simulink model "All_64_Coils"  (R2021b)
settings: Solver=FixedStepAuto  StartTime=-3000e-6  StopTime=3000e-6  FixedStep=25e-6  RelTol=1e-3
(part 11 of 70)

== system All_64_Coils/Coil-3/Inner_5_coils/Square-4 ==
  Input : Inport
  Input1 : Inport  Port=2
  Input2 : Inport  Port=3
  Input3 : Inport  Port=4
  F_xx_4 : Display
  F_xz_4 : Display
  F_yy_4 : Display
  F_yz_4 : Display
  Scope : Scope  NumInputPorts=4
  Square - 4 : MATLAB Function  TreatAsAtomicUnit=on
  Output : Outport
  Output1 : Outport  Port=2
  Output2 : Outport  Port=3
  Output3 : Outport  Port=4
  wires:
  Input3/1 -> Square - 4/4(I)
  Input/1 -> Square - 4/1(x)
  Input1/1 -> Square - 4/2(y)
  Input2/1 -> Square - 4/3(z)
  Square - 4/3(F_yy_4) -> F_yy_4/1, Output2/1, Scope/3
  Square - 4/2(F_xz_4) -> Scope/2, F_xz_4/1, Output1/1
  Square - 4/4(F_yz_4) -> F_yz_4/1, Output3/1, Scope/4
  Square - 4/1(F_xx_4) -> F_xx_4/1, Output/1, Scope/1

== system All_64_Coils/Coil-3/Inner_5_coils/Square-5 ==
  Input : Inport
  Input1 : Inport  Port=2
  Input2 : Inport  Port=3
  Input3 : Inport  Port=4
  F_xx_5 : Display
  F_xz_5 : Display
  F_yy_5 : Display
  F_yz_5 : Display
  Scope : Scope  NumInputPorts=4
  Square - 5 : MATLAB Function  TreatAsAtomicUnit=on
  Output : Outport
  Output1 : Outport  Port=2
  Output2 : Outport  Port=3
  Output3 : Outport  Port=4
  wires:
  Input3/1 -> Square - 5/4(I)
  Input/1 -> Square - 5/1(x)
  Input1/1 -> Square - 5/2(y)
  Input2/1 -> Square - 5/3(z)
  Square - 5/3(F_yy_5) -> F_yy_5/1, Output2/1, Scope/3
  Square - 5/2(F_xz_5) -> F_xz_5/1, Output1/1, Scope/2
  Square - 5/4(F_yz_5) -> F_yz_5/1, Output3/1, Scope/4
  Square - 5/1(F_xx_5) -> F_xx_5/1, Output/1, Scope/1

== system All_64_Coils/Coil-3/Outer_5_Coils/Square-10 ==
  Input : Inport
  Input1 : Inport  Port=2
  Input2 : Inport  Port=3
  Input3 : Inport  Port=4
  F_xx_10 : Display
  F_xz_10 : Display
  F_yy_10 : Display
  F_yz_10 : Display
  Scope : Scope  NumInputPorts=4
  Square - 10 : MATLAB Function  TreatAsAtomicUnit=on
  Output : Outport
  Output1 : Outport  Port=2
  Output2 : Outport  Port=3
  Output3 : Outport  Port=4
  wires:
  Square - 10/1(F_xx_10) -> Output/1, F_xx_10/1, Scope/1
  Square - 10/4(F_yz_10) -> Output3/1, F_yz_10/1, Scope/4
  Square - 10/2(F_xz_10) -> Output1/1, F_xz_10/1, Scope/2
  Square - 10/3(F_yy_10) -> Output2/1, F_yy_10/1, Scope/3
  Input2/1 -> Square - 10/3(z)
  Input1/1 -> Square - 10/2(y)
  Input/1 -> Square - 10/1(x)
  Input3/1 -> Square - 10/4(I)

== system All_64_Coils/Coil-3/Outer_5_Coils/Square-6 ==
  Input : Inport
  Input1 : Inport  Port=2
  Input2 : Inport  Port=3
  Input3 : Inport  Port=4
  F_xx_6 : Display
  F_xz_6 : Display
  F_yy_6 : Display
  F_yz_6 : Display
  Scope : Scope  NumInputPorts=4
  Square - 6 : MATLAB Function  TreatAsAtomicUnit=on
  Output : Outport
  Output1 : Outport  Port=2
  Output2 : Outport  Port=3
  Output3 : Outport  Port=4
  wires:
  Square - 6/2(F_xz_6) -> Output1/1, F_xz_6/1, Scope/2
  Square - 6/3(F_yy_6) -> F_yy_6/1, Output2/1, Scope/3
  Square - 6/1(F_xx_6) -> Output/1, F_xx_6/1, Scope/1
  Square - 6/4(F_yz_6) -> Output3/1, F_yz_6/1, Scope/4
  Input/1 -> Square - 6/1(x)
  Input1/1 -> Square - 6/2(y)
  Input2/1 -> Square - 6/3(z)
  Input3/1 -> Square - 6/4(I)

== system All_64_Coils/Coil-3/Outer_5_Coils/Square-7 ==
  Input : Inport
  Input1 : Inport  Port=2
  Input2 : Inport  Port=3
  Input3 : Inport  Port=4
  F_xx_7 : Display
  F_xz_7 : Display
  F_yy_7 : Display
  F_yz_7 : Display
  Scope : Scope  NumInputPorts=4
  Square - 7 : MATLAB Function  TreatAsAtomicUnit=on
  Output : Outport
  Output1 : Outport  Port=2
  Output2 : Outport  Port=3
  Output3 : Outport  Port=4
  wires:
  Square - 7/1(F_xx_7) -> Output/1, F_xx_7/1, Scope/1
  Square - 7/2(F_xz_7) -> Output1/1, F_xz_7/1, Scope/2
  Square - 7/4(F_yz_7) -> Output3/1, F_yz_7/1, Scope/4
  Square - 7/3(F_yy_7) -> Output2/1, F_yy_7/1, Scope/3
  Input2/1 -> Square - 7/3(z)
  Input1/1 -> Square - 7/2(y)
  Input/1 -> Square - 7/1(x)
  Input3/1 -> Square - 7/4(I)

== system All_64_Coils/Coil-3/Outer_5_Coils/Square-8 ==
  Input : Inport
  Input1 : Inport  Port=2
  Input2 : Inport  Port=3
  Input3 : Inport  Port=4
  F_xx_8 : Display
  F_xz_8 : Display
  F_yy_8 : Display
  F_yz_8 : Display
  Scope : Scope  NumInputPorts=4
  Square - 8 : MATLAB Function  TreatAsAtomicUnit=on
  Output : Outport
  Output1 : Outport  Port=2
  Output2 : Outport  Port=3
  Output3 : Outport  Port=4
  wires:
  Square - 8/1(F_xx_8) -> Output/1, F_xx_8/1, Scope/1
  Square - 8/4(F_yz_8) -> Output3/1, F_yz_8/1, Scope/4
  Square - 8/2(F_xz_8) -> Output1/1, F_xz_8/1, Scope/2
  Square - 8/3(F_yy_8) -> F_yy_8/1, Output2/1, Scope/3
  Input2/1 -> Square - 8/3(z)
  Input1/1 -> Square - 8/2(y)
  Input/1 -> Square - 8/1(x)
  Input3/1 -> Square - 8/4(I)

== system All_64_Coils/Coil-3/Outer_5_Coils/Square-9 ==
  Input : Inport
  Input1 : Inport  Port=2
  Input2 : Inport  Port=3
  Input3 : Inport  Port=4
  F_xx_9 : Display
  F_xz_9 : Display
  F_yy_9 : Display
  F_yz_9 : Display
  Scope : Scope  NumInputPorts=4
  Square - 9 : MATLAB Function  TreatAsAtomicUnit=on
  Output : Outport
  Output1 : Outport  Port=2
  Output2 : Outport  Port=3
  Output3 : Outport  Port=4
  wires:
  Square - 9/1(F_xx_9) -> Output/1, F_xx_9/1, Scope/1
  Square - 9/4(F_yz_9) -> Output3/1, F_yz_9/1, Scope/4
  Square - 9/2(F_xz_9) -> Output1/1, F_xz_9/1, Scope/2
  Square - 9/3(F_yy_9) -> Output2/1, F_yy_9/1, Scope/3
  Input2/1 -> Square - 9/3(z)
  Input1/1 -> Square - 9/2(y)
  Input/1 -> Square - 9/1(x)
  Input3/1 -> Square - 9/4(I)

== system All_64_Coils/Coil-30/Inner_5_coils/Square-1 ==
  Input : Inport
  Input1 : Inport  Port=2
  Input2 : Inport  Port=3
  Input3 : Inport  Port=4
  F_xx_1 : Display
  F_xz_1 : Display
  F_yy_1 : Display
  F_yz_1 : Display
  Scope : Scope  NumInputPorts=4
  Square - 1 : MATLAB Function  TreatAsAtomicUnit=on
  Output : Outport
  Output1 : Outport  Port=2
  Output2 : Outport  Port=3
  Output3 : Outport  Port=4
  wires:
  Input3/1 -> Square - 1/4(I)
  Input2/1 -> Square - 1/3(z)
  Input1/1 -> Square - 1/2(y)
  Input/1 -> Square - 1/1(x)
  Square - 1/4(F_yz_1) -> F_yz_1/1, Output3/1, Scope/4
  Square - 1/1(F_xx_1) -> Scope/1, F_xx_1/1, Output/1
  Square - 1/3(F_yy_1) -> Scope/3, Output2/1, F_yy_1/1
  Square - 1/2(F_xz_1) -> Scope/2, F_xz_1/1, Output1/1

== system All_64_Coils/Coil-30/Inner_5_coils/Square-2 ==
  Input : Inport
  Input1 : Inport  Port=2
  Input2 : Inport  Port=3
  Input3 : Inport  Port=4
  F_xx_2 : Display
  F_xz_2 : Display
  F_yy_2 : Display
  F_yz_2 : Display
  Scope : Scope  NumInputPorts=4
  Square - 2 : MATLAB Function  TreatAsAtomicUnit=on
  Output : Outport
  Output1 : Outport  Port=2
  Output2 : Outport  Port=3
  Output3 : Outport  Port=4
  wires:
  Input3/1 -> Square - 2/4(I)
  Input/1 -> Square - 2/1(x)
  Input1/1 -> Square - 2/2(y)
  Input2/1 -> Square - 2/3(z)
  Square - 2/3(F_yy_2) -> F_yy_2/1, Output2/1, Scope/3
  Square - 2/4(F_yz_2) -> F_yz_2/1, Output3/1, Scope/4
  Square - 2/2(F_xz_2) -> F_xz_2/1, Output1/1, Scope/2
  Square - 2/1(F_xx_2) -> F_xx_2/1, Output/1, Scope/1

== system All_64_Coils/Coil-30/Inner_5_coils/Square-3 ==
  Input : Inport
  Input1 : Inport  Port=2
  Input2 : Inport  Port=3
  Input3 : Inport  Port=4
  F_xx_3 : Display
  F_xz_3 : Display
  F_yy_3 : Display
  F_yz_3 : Display
  Scope : Scope  NumInputPorts=4
  Square - 3 : MATLAB Function  TreatAsAtomicUnit=on
  Output : Outport
  Output1 : Outport  Port=2
  Output2 : Outport  Port=3
  Output3 : Outport  Port=4
  wires:
  Input3/1 -> Square - 3/4(I)
  Input/1 -> Square - 3/1(x)
  Input1/1 -> Square - 3/2(y)
  Input2/1 -> Square - 3/3(z)
  Square - 3/3(F_yy_3) -> Output2/1, F_yy_3/1, Scope/3
  Square - 3/2(F_xz_3) -> F_xz_3/1, Output1/1, Scope/2
  Square - 3/4(F_yz_3) -> F_yz_3/1, Output3/1, Scope/4
  Square - 3/1(F_xx_3) -> F_xx_3/1, Output/1, Scope/1

== system All_64_Coils/Coil-30/Inner_5_coils/Square-4 ==
  Input : Inport
  Input1 : Inport  Port=2
  Input2 : Inport  Port=3
  Input3 : Inport  Port=4
  F_xx_4 : Display
  F_xz_4 : Display
  F_yy_4 : Display
  F_yz_4 : Display
  Scope : Scope  NumInputPorts=4
  Square - 4 : MATLAB Function  TreatAsAtomicUnit=on
  Output : Outport
  Output1 : Outport  Port=2
  Output2 : Outport  Port=3
  Output3 : Outport  Port=4
  wires:
  Input3/1 -> Square - 4/4(I)
  Input/1 -> Square - 4/1(x)
  Input1/1 -> Square - 4/2(y)
  Input2/1 -> Square - 4/3(z)
  Square - 4/3(F_yy_4) -> F_yy_4/1, Output2/1, Scope/3
  Square - 4/2(F_xz_4) -> Scope/2, F_xz_4/1, Output1/1
  Square - 4/4(F_yz_4) -> F_yz_4/1, Output3/1, Scope/4
  Square - 4/1(F_xx_4) -> F_xx_4/1, Output/1, Scope/1

== system All_64_Coils/Coil-30/Inner_5_coils/Square-5 ==
  Input : Inport
  Input1 : Inport  Port=2
  Input2 : Inport  Port=3
  Input3 : Inport  Port=4
  F_xx_5 : Display
  F_xz_5 : Display
  F_yy_5 : Display
  F_yz_5 : Display
  Scope : Scope  NumInputPorts=4
  Square - 5 : MATLAB Function  TreatAsAtomicUnit=on
  Output : Outport
  Output1 : Outport  Port=2
  Output2 : Outport  Port=3
  Output3 : Outport  Port=4
  wires:
  Input3/1 -> Square - 5/4(I)
  Input/1 -> Square - 5/1(x)
  Input1/1 -> Square - 5/2(y)
  Input2/1 -> Square - 5/3(z)
  Square - 5/3(F_yy_5) -> F_yy_5/1, Output2/1, Scope/3
  Square - 5/2(F_xz_5) -> F_xz_5/1, Output1/1, Scope/2
  Square - 5/4(F_yz_5) -> F_yz_5/1, Output3/1, Scope/4
  Square - 5/1(F_xx_5) -> F_xx_5/1, Output/1, Scope/1

== system All_64_Coils/Coil-30/Outer_5_Coils/Square-10 ==
  Input : Inport
  Input1 : Inport  Port=2
  Input2 : Inport  Port=3
  Input3 : Inport  Port=4
  F_xx_10 : Display
  F_xz_10 : Display
  F_yy_10 : Display
  F_yz_10 : Display
  Scope : Scope  NumInputPorts=4
  Square - 10 : MATLAB Function  TreatAsAtomicUnit=on
  Output : Outport
  Output1 : Outport  Port=2
  Output2 : Outport  Port=3
  Output3 : Outport  Port=4
  wires:
  Square - 10/1(F_xx_10) -> Output/1, F_xx_10/1, Scope/1
  Square - 10/4(F_yz_10) -> Output3/1, F_yz_10/1, Scope/4
  Square - 10/2(F_xz_10) -> Output1/1, F_xz_10/1, Scope/2
  Square - 10/3(F_yy_10) -> Output2/1, F_yy_10/1, Scope/3
  Input2/1 -> Square - 10/3(z)
  Input1/1 -> Square - 10/2(y)
  Input/1 -> Square - 10/1(x)
  Input3/1 -> Square - 10/4(I)

== system All_64_Coils/Coil-30/Outer_5_Coils/Square-6 ==
  Input : Inport
  Input1 : Inport  Port=2
  Input2 : Inport  Port=3
  Input3 : Inport  Port=4
  F_xx_6 : Display
  F_xz_6 : Display
  F_yy_6 : Display
  F_yz_6 : Display
  Scope : Scope  NumInputPorts=4
  Square - 6 : MATLAB Function  TreatAsAtomicUnit=on
  Output : Outport
  Output1 : Outport  Port=2
  Output2 : Outport  Port=3
  Output3 : Outport  Port=4
  wires:
  Square - 6/2(F_xz_6) -> Output1/1, F_xz_6/1, Scope/2
  Square - 6/3(F_yy_6) -> F_yy_6/1, Output2/1, Scope/3
  Square - 6/1(F_xx_6) -> Output/1, F_xx_6/1, Scope/1
  Square - 6/4(F_yz_6) -> Output3/1, F_yz_6/1, Scope/4
  Input/1 -> Square - 6/1(x)
  Input1/1 -> Square - 6/2(y)
  Input2/1 -> Square - 6/3(z)
  Input3/1 -> Square - 6/4(I)

== system All_64_Coils/Coil-30/Outer_5_Coils/Square-7 ==
  Input : Inport
  Input1 : Inport  Port=2
  Input2 : Inport  Port=3
  Input3 : Inport  Port=4
  F_xx_7 : Display
  F_xz_7 : Display
  F_yy_7 : Display
  F_yz_7 : Display
  Scope : Scope  NumInputPorts=4
  Square - 7 : MATLAB Function  TreatAsAtomicUnit=on
  Output : Outport
  Output1 : Outport  Port=2
  Output2 : Outport  Port=3
  Output3 : Outport  Port=4
  wires:
  Square - 7/1(F_xx_7) -> Output/1, F_xx_7/1, Scope/1
  Square - 7/2(F_xz_7) -> Output1/1, F_xz_7/1, Scope/2
  Square - 7/4(F_yz_7) -> Output3/1, F_yz_7/1, Scope/4
  Square - 7/3(F_yy_7) -> Output2/1, F_yy_7/1, Scope/3
  Input2/1 -> Square - 7/3(z)
  Input1/1 -> Square - 7/2(y)
  Input/1 -> Square - 7/1(x)
  Input3/1 -> Square - 7/4(I)

== system All_64_Coils/Coil-30/Outer_5_Coils/Square-8 ==
  Input : Inport
  Input1 : Inport  Port=2
  Input2 : Inport  Port=3
  Input3 : Inport  Port=4
  F_xx_8 : Display
  F_xz_8 : Display
  F_yy_8 : Display
  F_yz_8 : Display
  Scope : Scope  NumInputPorts=4
  Square - 8 : MATLAB Function  TreatAsAtomicUnit=on
  Output : Outport
  Output1 : Outport  Port=2
  Output2 : Outport  Port=3
  Output3 : Outport  Port=4
  wires:
  Square - 8/1(F_xx_8) -> Output/1, F_xx_8/1, Scope/1
  Square - 8/4(F_yz_8) -> Output3/1, F_yz_8/1, Scope/4
  Square - 8/2(F_xz_8) -> Output1/1, F_xz_8/1, Scope/2
  Square - 8/3(F_yy_8) -> F_yy_8/1, Output2/1, Scope/3
  Input2/1 -> Square - 8/3(z)
  Input1/1 -> Square - 8/2(y)
  Input/1 -> Square - 8/1(x)
  Input3/1 -> Square - 8/4(I)

== system All_64_Coils/Coil-30/Outer_5_Coils/Square-9 ==
  Input : Inport
  Input1 : Inport  Port=2
  Input2 : Inport  Port=3
  Input3 : Inport  Port=4
  F_xx_9 : Display
  F_xz_9 : Display
  F_yy_9 : Display
  F_yz_9 : Display
  Scope : Scope  NumInputPorts=4
  Square - 9 : MATLAB Function  TreatAsAtomicUnit=on
  Output : Outport
  Output1 : Outport  Port=2
  Output2 : Outport  Port=3
  Output3 : Outport  Port=4
  wires:
  Square - 9/1(F_xx_9) -> Output/1, F_xx_9/1, Scope/1
  Square - 9/4(F_yz_9) -> Output3/1, F_yz_9/1, Scope/4
  Square - 9/2(F_xz_9) -> Output1/1, F_xz_9/1, Scope/2
  Square - 9/3(F_yy_9) -> Output2/1, F_yy_9/1, Scope/3
  Input2/1 -> Square - 9/3(z)
  Input1/1 -> Square - 9/2(y)
  Input/1 -> Square - 9/1(x)
  Input3/1 -> Square - 9/4(I)

== system All_64_Coils/Coil-31/Inner_5_coils/Square-1 ==
  Input : Inport
  Input1 : Inport  Port=2
  Input2 : Inport  Port=3
  Input3 : Inport  Port=4
  F_xx_1 : Display
  F_xz_1 : Display
  F_yy_1 : Display
  F_yz_1 : Display
  Scope : Scope  NumInputPorts=4
  Square - 1 : MATLAB Function  TreatAsAtomicUnit=on
  Output : Outport
  Output1 : Outport  Port=2
  Output2 : Outport  Port=3
  Output3 : Outport  Port=4
  wires:
  Square - 1/2(F_xz_1) -> Output1/1, F_xz_1/1, Scope/2
  Square - 1/3(F_yy_1) -> F_yy_1/1, Output2/1, Scope/3
  Square - 1/1(F_xx_1) -> Output/1, F_xx_1/1, Scope/1
  Square - 1/4(F_yz_1) -> Scope/4, Output3/1, F_yz_1/1
  Input/1 -> Square - 1/1(x)
  Input1/1 -> Square - 1/2(y)
  Input2/1 -> Square - 1/3(z)
  Input3/1 -> Square - 1/4(I)

== system All_64_Coils/Coil-31/Inner_5_coils/Square-2 ==
  Input : Inport
  Input1 : Inport  Port=2
  Input2 : Inport  Port=3
  Input3 : Inport  Port=4
  F_xx_2 : Display
  F_xz_2 : Display
  F_yy_2 : Display
  F_yz_2 : Display
  Scope : Scope  NumInputPorts=4
  Square - 2 : MATLAB Function  TreatAsAtomicUnit=on
  Output : Outport
  Output1 : Outport  Port=2
  Output2 : Outport  Port=3
  Output3 : Outport  Port=4
  wires:
  Square - 2/1(F_xx_2) -> Scope/1, Output/1, F_xx_2/1
  Square - 2/2(F_xz_2) -> Scope/2, Output1/1, F_xz_2/1
  Square - 2/4(F_yz_2) -> Scope/4, Output3/1, F_yz_2/1
  Square - 2/3(F_yy_2) -> Scope/3, Output2/1, F_yy_2/1
  Input2/1 -> Square - 2/3(z)
  Input1/1 -> Square - 2/2(y)
  Input/1 -> Square - 2/1(x)
  Input3/1 -> Square - 2/4(I)

== system All_64_Coils/Coil-31/Inner_5_coils/Square-3 ==
  Input : Inport
  Input1 : Inport  Port=2
  Input2 : Inport  Port=3
  Input3 : Inport  Port=4
  F_xx_3 : Display
  F_xz_3 : Display
  F_yy_3 : Display
  F_yz_3 : Display
  Scope : Scope  NumInputPorts=4
  Square - 3 : MATLAB Function  TreatAsAtomicUnit=on
  Output : Outport
  Output1 : Outport  Port=2
  Output2 : Outport  Port=3
  Output3 : Outport  Port=4
  wires:
  Square - 3/1(F_xx_3) -> Scope/1, Output/1, F_xx_3/1
  Square - 3/4(F_yz_3) -> Scope/4, Output3/1, F_yz_3/1
  Square - 3/2(F_xz_3) -> Scope/2, Output1/1, F_xz_3/1
  Square - 3/3(F_yy_3) -> Scope/3, F_yy_3/1, Output2/1
  Input2/1 -> Square - 3/3(z)
  Input1/1 -> Square - 3/2(y)
  Input/1 -> Square - 3/1(x)
  Input3/1 -> Square - 3/4(I)

== system All_64_Coils/Coil-31/Inner_5_coils/Square-4 ==
  Input : Inport
  Input1 : Inport  Port=2
  Input2 : Inport  Port=3
  Input3 : Inport  Port=4
  F_xx_4 : Display
  F_xz_4 : Display
  F_yy_4 : Display
  F_yz_4 : Display
  Scope : Scope  NumInputPorts=4
  Square - 4 : MATLAB Function  TreatAsAtomicUnit=on
  Output : Outport
  Output1 : Outport  Port=2
  Output2 : Outport  Port=3
  Output3 : Outport  Port=4
  wires:
  Square - 4/1(F_xx_4) -> Scope/1, Output/1, F_xx_4/1
  Square - 4/4(F_yz_4) -> Scope/4, Output3/1, F_yz_4/1
  Square - 4/2(F_xz_4) -> Output1/1, F_xz_4/1, Scope/2
  Square - 4/3(F_yy_4) -> Scope/3, Output2/1, F_yy_4/1
  Input2/1 -> Square - 4/3(z)
  Input1/1 -> Square - 4/2(y)
  Input/1 -> Square - 4/1(x)
  Input3/1 -> Square - 4/4(I)

== system All_64_Coils/Coil-31/Inner_5_coils/Square-5 ==
  Input : Inport
  Input1 : Inport  Port=2
  Input2 : Inport  Port=3
  Input3 : Inport  Port=4
  F_xx_5 : Display
  F_xz_5 : Display
  F_yy_5 : Display
  F_yz_5 : Display
  Scope : Scope  NumInputPorts=4
  Square - 5 : MATLAB Function  TreatAsAtomicUnit=on
  Output : Outport
  Output1 : Outport  Port=2
  Output2 : Outport  Port=3
  Output3 : Outport  Port=4
  wires:
  Square - 5/1(F_xx_5) -> Scope/1, Output/1, F_xx_5/1
  Square - 5/4(F_yz_5) -> Scope/4, Output3/1, F_yz_5/1
  Square - 5/2(F_xz_5) -> Scope/2, Output1/1, F_xz_5/1
  Square - 5/3(F_yy_5) -> Scope/3, Output2/1, F_yy_5/1
  Input2/1 -> Square - 5/3(z)
  Input1/1 -> Square - 5/2(y)
  Input/1 -> Square - 5/1(x)
  Input3/1 -> Square - 5/4(I)

== system All_64_Coils/Coil-31/Outer_5_Coils/Square-10 ==
  Input : Inport
  Input1 : Inport  Port=2
  Input2 : Inport  Port=3
  Input3 : Inport  Port=4
  F_xx_10 : Display
  F_xz_10 : Display
  F_yy_10 : Display
  F_yz_10 : Display
  Scope : Scope  NumInputPorts=4
  Square - 10 : MATLAB Function  TreatAsAtomicUnit=on
  Output : Outport
  Output1 : Outport  Port=2
  Output2 : Outport  Port=3
  Output3 : Outport  Port=4
  wires:
  Input3/1 -> Square - 10/4(I)
  Input/1 -> Square - 10/1(x)
  Input1/1 -> Square - 10/2(y)
  Input2/1 -> Square - 10/3(z)
  Square - 10/3(F_yy_10) -> Scope/3, F_yy_10/1, Output2/1
  Square - 10/2(F_xz_10) -> Scope/2, F_xz_10/1, Output1/1
  Square - 10/4(F_yz_10) -> Scope/4, F_yz_10/1, Output3/1
  Square - 10/1(F_xx_10) -> Scope/1, F_xx_10/1, Output/1

== system All_64_Coils/Coil-31/Outer_5_Coils/Square-6 ==
  Input : Inport
  Input1 : Inport  Port=2
  Input2 : Inport  Port=3
  Input3 : Inport  Port=4
  F_xx_6 : Display
  F_xz_6 : Display
  F_yy_6 : Display
  F_yz_6 : Display
  Scope : Scope  NumInputPorts=4
  Square - 6 : MATLAB Function  TreatAsAtomicUnit=on
  Output : Outport
  Output1 : Outport  Port=2
  Output2 : Outport  Port=3
  Output3 : Outport  Port=4
  wires:
  Input3/1 -> Square - 6/4(I)
  Input2/1 -> Square - 6/3(z)
  Input1/1 -> Square - 6/2(y)
  Input/1 -> Square - 6/1(x)
  Square - 6/4(F_yz_6) -> Scope/4, F_yz_6/1, Output3/1
  Square - 6/1(F_xx_6) -> Scope/1, F_xx_6/1, Output/1
  Square - 6/3(F_yy_6) -> Scope/3, Output2/1, F_yy_6/1
  Square - 6/2(F_xz_6) -> Scope/2, F_xz_6/1, Output1/1

== system All_64_Coils/Coil-31/Outer_5_Coils/Square-7 ==
  Input : Inport
  Input1 : Inport  Port=2
  Input2 : Inport  Port=3
  Input3 : Inport  Port=4
  F_xx_7 : Display
  F_xz_7 : Display
  F_yy_7 : Display
  F_yz_7 : Display
  Scope : Scope  NumInputPorts=4
  Square - 7 : MATLAB Function  TreatAsAtomicUnit=on
  Output : Outport
  Output1 : Outport  Port=2
  Output2 : Outport  Port=3
  Output3 : Outport  Port=4
  wires:
  Input3/1 -> Square - 7/4(I)
  Input/1 -> Square - 7/1(x)
  Input1/1 -> Square - 7/2(y)
  Input2/1 -> Square - 7/3(z)
  Square - 7/3(F_yy_7) -> Scope/3, F_yy_7/1, Output2/1
  Square - 7/4(F_yz_7) -> Scope/4, F_yz_7/1, Output3/1
  Square - 7/2(F_xz_7) -> Scope/2, F_xz_7/1, Output1/1
  Square - 7/1(F_xx_7) -> Scope/1, F_xx_7/1, Output/1

== system All_64_Coils/Coil-31/Outer_5_Coils/Square-8 ==
  Input : Inport
  Input1 : Inport  Port=2
  Input2 : Inport  Port=3
  Input3 : Inport  Port=4
  F_xx_8 : Display
  F_xz_8 : Display
  F_yy_8 : Display
  F_yz_8 : Display
  Scope : Scope  NumInputPorts=4
  Square - 8 : MATLAB Function  TreatAsAtomicUnit=on
  Output : Outport
  Output1 : Outport  Port=2
  Output2 : Outport  Port=3
  Output3 : Outport  Port=4
  wires:
  Input3/1 -> Square - 8/4(I)
  Input/1 -> Square - 8/1(x)
  Input1/1 -> Square - 8/2(y)
  Input2/1 -> Square - 8/3(z)
  Square - 8/3(F_yy_8) -> Scope/3, Output2/1, F_yy_8/1
  Square - 8/2(F_xz_8) -> Scope/2, F_xz_8/1, Output1/1
  Square - 8/4(F_yz_8) -> Scope/4, F_yz_8/1, Output3/1
  Square - 8/1(F_xx_8) -> Scope/1, F_xx_8/1, Output/1

== system All_64_Coils/Coil-31/Outer_5_Coils/Square-9 ==
  Input : Inport
  Input1 : Inport  Port=2
  Input2 : Inport  Port=3
  Input3 : Inport  Port=4
  F_xx_9 : Display
  F_xz_9 : Display
  F_yy_9 : Display
  F_yz_9 : Display
  Scope : Scope  NumInputPorts=4
  Square - 9 : MATLAB Function  TreatAsAtomicUnit=on
  Output : Outport
  Output1 : Outport  Port=2
  Output2 : Outport  Port=3
  Output3 : Outport  Port=4
  wires:
  Input3/1 -> Square - 9/4(I)
  Input/1 -> Square - 9/1(x)
  Input1/1 -> Square - 9/2(y)
  Input2/1 -> Square - 9/3(z)
  Square - 9/3(F_yy_9) -> Scope/3, F_yy_9/1, Output2/1
  Square - 9/2(F_xz_9) -> Scope/2, F_xz_9/1, Output1/1
  Square - 9/4(F_yz_9) -> Scope/4, F_yz_9/1, Output3/1
  Square - 9/1(F_xx_9) -> Scope/1, F_xx_9/1, Output/1

== system All_64_Coils/Coil-32/Inner_5_coils/Square-1 ==
  Input : Inport
  Input1 : Inport  Port=2
  Input2 : Inport  Port=3
  Input3 : Inport  Port=4
  F_xx_1 : Display
  F_xz_1 : Display
  F_yy_1 : Display
  F_yz_1 : Display
  Scope : Scope  NumInputPorts=4
  Square - 1 : MATLAB Function  TreatAsAtomicUnit=on
  Output : Outport
  Output1 : Outport  Port=2
  Output2 : Outport  Port=3
  Output3 : Outport  Port=4
  wires:
  Square - 1/2(F_xz_1) -> Output1/1, F_xz_1/1, Scope/2
  Square - 1/3(F_yy_1) -> F_yy_1/1, Output2/1, Scope/3
  Square - 1/1(F_xx_1) -> Output/1, F_xx_1/1, Scope/1
  Square - 1/4(F_yz_1) -> Scope/4, Output3/1, F_yz_1/1
  Input/1 -> Square - 1/1(x)
  Input1/1 -> Square - 1/2(y)
  Input2/1 -> Square - 1/3(z)
  Input3/1 -> Square - 1/4(I)

== system All_64_Coils/Coil-32/Inner_5_coils/Square-2 ==
  Input : Inport
  Input1 : Inport  Port=2
  Input2 : Inport  Port=3
  Input3 : Inport  Port=4
  F_xx_2 : Display
  F_xz_2 : Display
  F_yy_2 : Display
  F_yz_2 : Display
  Scope : Scope  NumInputPorts=4
  Square - 2 : MATLAB Function  TreatAsAtomicUnit=on
  Output : Outport
  Output1 : Outport  Port=2
  Output2 : Outport  Port=3
  Output3 : Outport  Port=4
  wires:
  Square - 2/1(F_xx_2) -> Scope/1, Output/1, F_xx_2/1
  Square - 2/2(F_xz_2) -> Scope/2, Output1/1, F_xz_2/1
  Square - 2/4(F_yz_2) -> Scope/4, Output3/1, F_yz_2/1
  Square - 2/3(F_yy_2) -> Scope/3, Output2/1, F_yy_2/1
  Input2/1 -> Square - 2/3(z)
  Input1/1 -> Square - 2/2(y)
  Input/1 -> Square - 2/1(x)
  Input3/1 -> Square - 2/4(I)

== system All_64_Coils/Coil-32/Inner_5_coils/Square-3 ==
  Input : Inport
  Input1 : Inport  Port=2
  Input2 : Inport  Port=3
  Input3 : Inport  Port=4
  F_xx_3 : Display
  F_xz_3 : Display
  F_yy_3 : Display
  F_yz_3 : Display
  Scope : Scope  NumInputPorts=4
  Square - 3 : MATLAB Function  TreatAsAtomicUnit=on
  Output : Outport
  Output1 : Outport  Port=2
  Output2 : Outport  Port=3
  Output3 : Outport  Port=4
  wires:
  Square - 3/1(F_xx_3) -> Scope/1, Output/1, F_xx_3/1
  Square - 3/4(F_yz_3) -> Scope/4, Output3/1, F_yz_3/1
  Square - 3/2(F_xz_3) -> Scope/2, Output1/1, F_xz_3/1
  Square - 3/3(F_yy_3) -> Scope/3, F_yy_3/1, Output2/1
  Input2/1 -> Square - 3/3(z)
  Input1/1 -> Square - 3/2(y)
  Input/1 -> Square - 3/1(x)
  Input3/1 -> Square - 3/4(I)

== system All_64_Coils/Coil-32/Inner_5_coils/Square-4 ==
  Input : Inport
  Input1 : Inport  Port=2
  Input2 : Inport  Port=3
  Input3 : Inport  Port=4
  F_xx_4 : Display
  F_xz_4 : Display
  F_yy_4 : Display
  F_yz_4 : Display
  Scope : Scope  NumInputPorts=4
  Square - 4 : MATLAB Function  TreatAsAtomicUnit=on
  Output : Outport
  Output1 : Outport  Port=2
  Output2 : Outport  Port=3
  Output3 : Outport  Port=4
  wires:
  Square - 4/1(F_xx_4) -> Scope/1, Output/1, F_xx_4/1
  Square - 4/4(F_yz_4) -> Scope/4, Output3/1, F_yz_4/1
  Square - 4/2(F_xz_4) -> Output1/1, F_xz_4/1, Scope/2
  Square - 4/3(F_yy_4) -> Scope/3, Output2/1, F_yy_4/1
  Input2/1 -> Square - 4/3(z)
  Input1/1 -> Square - 4/2(y)
  Input/1 -> Square - 4/1(x)
  Input3/1 -> Square - 4/4(I)

== system All_64_Coils/Coil-32/Inner_5_coils/Square-5 ==
  Input : Inport
  Input1 : Inport  Port=2
  Input2 : Inport  Port=3
  Input3 : Inport  Port=4
  F_xx_5 : Display
  F_xz_5 : Display
  F_yy_5 : Display
  F_yz_5 : Display
  Scope : Scope  NumInputPorts=4
  Square - 5 : MATLAB Function  TreatAsAtomicUnit=on
  Output : Outport
  Output1 : Outport  Port=2
  Output2 : Outport  Port=3
  Output3 : Outport  Port=4
  wires:
  Square - 5/1(F_xx_5) -> Scope/1, Output/1, F_xx_5/1
  Square - 5/4(F_yz_5) -> Scope/4, Output3/1, F_yz_5/1
  Square - 5/2(F_xz_5) -> Scope/2, Output1/1, F_xz_5/1
  Square - 5/3(F_yy_5) -> Scope/3, Output2/1, F_yy_5/1
  Input2/1 -> Square - 5/3(z)
  Input1/1 -> Square - 5/2(y)
  Input/1 -> Square - 5/1(x)
  Input3/1 -> Square - 5/4(I)

== system All_64_Coils/Coil-32/Outer_5_Coils/Square-10 ==
  Input : Inport
  Input1 : Inport  Port=2
  Input2 : Inport  Port=3
  Input3 : Inport  Port=4
  F_xx_10 : Display
  F_xz_10 : Display
  F_yy_10 : Display
  F_yz_10 : Display
  Scope : Scope  NumInputPorts=4
  Square - 10 : MATLAB Function  TreatAsAtomicUnit=on
  Output : Outport
  Output1 : Outport  Port=2
  Output2 : Outport  Port=3
  Output3 : Outport  Port=4
  wires:
  Input3/1 -> Square - 10/4(I)
  Input/1 -> Square - 10/1(x)
  Input1/1 -> Square - 10/2(y)
  Input2/1 -> Square - 10/3(z)
  Square - 10/3(F_yy_10) -> Scope/3, F_yy_10/1, Output2/1
  Square - 10/2(F_xz_10) -> Scope/2, F_xz_10/1, Output1/1
  Square - 10/4(F_yz_10) -> Scope/4, F_yz_10/1, Output3/1
  Square - 10/1(F_xx_10) -> Scope/1, F_xx_10/1, Output/1

== system All_64_Coils/Coil-32/Outer_5_Coils/Square-6 ==
  Input : Inport
  Input1 : Inport  Port=2
  Input2 : Inport  Port=3
  Input3 : Inport  Port=4
  F_xx_6 : Display
  F_xz_6 : Display
  F_yy_6 : Display
  F_yz_6 : Display
  Scope : Scope  NumInputPorts=4
  Square - 6 : MATLAB Function  TreatAsAtomicUnit=on
  Output : Outport
  Output1 : Outport  Port=2
  Output2 : Outport  Port=3
  Output3 : Outport  Port=4
  wires:
  Input3/1 -> Square - 6/4(I)
  Input2/1 -> Square - 6/3(z)
  Input1/1 -> Square - 6/2(y)
  Input/1 -> Square - 6/1(x)
  Square - 6/4(F_yz_6) -> Scope/4, F_yz_6/1, Output3/1
  Square - 6/1(F_xx_6) -> Scope/1, F_xx_6/1, Output/1
  Square - 6/3(F_yy_6) -> Scope/3, Output2/1, F_yy_6/1
  Square - 6/2(F_xz_6) -> Scope/2, F_xz_6/1, Output1/1

== system All_64_Coils/Coil-32/Outer_5_Coils/Square-7 ==
  Input : Inport
  Input1 : Inport  Port=2
  Input2 : Inport  Port=3
  Input3 : Inport  Port=4
  F_xx_7 : Display
  F_xz_7 : Display
  F_yy_7 : Display
  F_yz_7 : Display
  Scope : Scope  NumInputPorts=4
  Square - 7 : MATLAB Function  TreatAsAtomicUnit=on
  Output : Outport
  Output1 : Outport  Port=2
  Output2 : Outport  Port=3
  Output3 : Outport  Port=4
  wires:
  Input3/1 -> Square - 7/4(I)
  Input/1 -> Square - 7/1(x)
  Input1/1 -> Square - 7/2(y)
  Input2/1 -> Square - 7/3(z)
  Square - 7/3(F_yy_7) -> Scope/3, F_yy_7/1, Output2/1
  Square - 7/4(F_yz_7) -> Scope/4, F_yz_7/1, Output3/1
  Square - 7/2(F_xz_7) -> Scope/2, F_xz_7/1, Output1/1
  Square - 7/1(F_xx_7) -> Scope/1, F_xx_7/1, Output/1

== system All_64_Coils/Coil-32/Outer_5_Coils/Square-8 ==
  Input : Inport
  Input1 : Inport  Port=2
  Input2 : Inport  Port=3
  Input3 : Inport  Port=4
  F_xx_8 : Display
  F_xz_8 : Display
  F_yy_8 : Display
  F_yz_8 : Display
  Scope : Scope  NumInputPorts=4
  Square - 8 : MATLAB Function  TreatAsAtomicUnit=on
  Output : Outport
  Output1 : Outport  Port=2
  Output2 : Outport  Port=3
  Output3 : Outport  Port=4
  wires:
  Input3/1 -> Square - 8/4(I)
  Input/1 -> Square - 8/1(x)
  Input1/1 -> Square - 8/2(y)
  Input2/1 -> Square - 8/3(z)
  Square - 8/3(F_yy_8) -> Scope/3, Output2/1, F_yy_8/1
  Square - 8/2(F_xz_8) -> Scope/2, F_xz_8/1, Output1/1
  Square - 8/4(F_yz_8) -> Scope/4, F_yz_8/1, Output3/1
  Square - 8/1(F_xx_8) -> Scope/1, F_xx_8/1, Output/1

== system All_64_Coils/Coil-32/Outer_5_Coils/Square-9 ==
  Input : Inport
  Input1 : Inport  Port=2
  Input2 : Inport  Port=3
  Input3 : Inport  Port=4
  F_xx_9 : Display
  F_xz_9 : Display
  F_yy_9 : Display
  F_yz_9 : Display
  Scope : Scope  NumInputPorts=4
  Square - 9 : MATLAB Function  TreatAsAtomicUnit=on
  Output : Outport
  Output1 : Outport  Port=2
  Output2 : Outport  Port=3
  Output3 : Outport  Port=4
  wires:
  Input3/1 -> Square - 9/4(I)
  Input/1 -> Square - 9/1(x)
  Input1/1 -> Square - 9/2(y)
  Input2/1 -> Square - 9/3(z)
  Square - 9/3(F_yy_9) -> Scope/3, F_yy_9/1, Output2/1
  Square - 9/2(F_xz_9) -> Scope/2, F_xz_9/1, Output1/1
  Square - 9/4(F_yz_9) -> Scope/4, F_yz_9/1, Output3/1
  Square - 9/1(F_xx_9) -> Scope/1, F_xx_9/1, Output/1

== system All_64_Coils/Coil-33/Inner_5_coils/Square-1 ==
  Input : Inport
  Input1 : Inport  Port=2
  Input2 : Inport  Port=3
  Input3 : Inport  Port=4
  F_xx_1 : Display
  F_xz_1 : Display
  F_yy_1 : Display
  F_yz_1 : Display
  Scope : Scope  NumInputPorts=4
  Square - 1 : MATLAB Function  TreatAsAtomicUnit=on
  Output : Outport
  Output1 : Outport  Port=2
  Output2 : Outport  Port=3
  Output3 : Outport  Port=4
  wires:
  Input3/1 -> Square - 1/4(I)
  Input2/1 -> Square - 1/3(z)
  Input1/1 -> Square - 1/2(y)
  Input/1 -> Square - 1/1(x)
  Square - 1/4(F_yz_1) -> F_yz_1/1, Output3/1, Scope/4
  Square - 1/1(F_xx_1) -> Scope/1, F_xx_1/1, Output/1
  Square - 1/3(F_yy_1) -> Scope/3, Output2/1, F_yy_1/1
  Square - 1/2(F_xz_1) -> Scope/2, F_xz_1/1, Output1/1

== system All_64_Coils/Coil-33/Inner_5_coils/Square-2 ==
  Input : Inport
  Input1 : Inport  Port=2
  Input2 : Inport  Port=3
  Input3 : Inport  Port=4
  F_xx_2 : Display
  F_xz_2 : Display
  F_yy_2 : Display
  F_yz_2 : Display
  Scope : Scope  NumInputPorts=4
  Square - 2 : MATLAB Function  TreatAsAtomicUnit=on
  Output : Outport
  Output1 : Outport  Port=2
  Output2 : Outport  Port=3
  Output3 : Outport  Port=4
  wires:
  Input3/1 -> Square - 2/4(I)
  Input/1 -> Square - 2/1(x)
  Input1/1 -> Square - 2/2(y)
  Input2/1 -> Square - 2/3(z)
  Square - 2/3(F_yy_2) -> F_yy_2/1, Output2/1, Scope/3
  Square - 2/4(F_yz_2) -> F_yz_2/1, Output3/1, Scope/4
  Square - 2/2(F_xz_2) -> F_xz_2/1, Output1/1, Scope/2
  Square - 2/1(F_xx_2) -> F_xx_2/1, Output/1, Scope/1

== system All_64_Coils/Coil-33/Inner_5_coils/Square-3 ==
  Input : Inport
  Input1 : Inport  Port=2
  Input2 : Inport  Port=3
  Input3 : Inport  Port=4
  F_xx_3 : Display
  F_xz_3 : Display
  F_yy_3 : Display
  F_yz_3 : Display
  Scope : Scope  NumInputPorts=4
  Square - 3 : MATLAB Function  TreatAsAtomicUnit=on
  Output : Outport
  Output1 : Outport  Port=2
  Output2 : Outport  Port=3
  Output3 : Outport  Port=4
  wires:
  Input3/1 -> Square - 3/4(I)
  Input/1 -> Square - 3/1(x)
  Input1/1 -> Square - 3/2(y)
  Input2/1 -> Square - 3/3(z)
  Square - 3/3(F_yy_3) -> Output2/1, F_yy_3/1, Scope/3
  Square - 3/2(F_xz_3) -> F_xz_3/1, Output1/1, Scope/2
  Square - 3/4(F_yz_3) -> F_yz_3/1, Output3/1, Scope/4
  Square - 3/1(F_xx_3) -> F_xx_3/1, Output/1, Scope/1

== system All_64_Coils/Coil-33/Inner_5_coils/Square-4 ==
  Input : Inport
  Input1 : Inport  Port=2
  Input2 : Inport  Port=3
  Input3 : Inport  Port=4
  F_xx_4 : Display
  F_xz_4 : Display
  F_yy_4 : Display
  F_yz_4 : Display
  Scope : Scope  NumInputPorts=4
  Square - 4 : MATLAB Function  TreatAsAtomicUnit=on
  Output : Outport
  Output1 : Outport  Port=2
  Output2 : Outport  Port=3
  Output3 : Outport  Port=4
  wires:
  Input3/1 -> Square - 4/4(I)
  Input/1 -> Square - 4/1(x)
  Input1/1 -> Square - 4/2(y)
  Input2/1 -> Square - 4/3(z)
  Square - 4/3(F_yy_4) -> F_yy_4/1, Output2/1, Scope/3
  Square - 4/2(F_xz_4) -> Scope/2, F_xz_4/1, Output1/1
  Square - 4/4(F_yz_4) -> F_yz_4/1, Output3/1, Scope/4
  Square - 4/1(F_xx_4) -> F_xx_4/1, Output/1, Scope/1

== system All_64_Coils/Coil-33/Inner_5_coils/Square-5 ==
  Input : Inport
  Input1 : Inport  Port=2
  Input2 : Inport  Port=3
  Input3 : Inport  Port=4
  F_xx_5 : Display
  F_xz_5 : Display
  F_yy_5 : Display
  F_yz_5 : Display
  Scope : Scope  NumInputPorts=4
  Square - 5 : MATLAB Function  TreatAsAtomicUnit=on
  Output : Outport
  Output1 : Outport  Port=2
  Output2 : Outport  Port=3
  Output3 : Outport  Port=4
  wires:
  Input3/1 -> Square - 5/4(I)
  Input/1 -> Square - 5/1(x)
  Input1/1 -> Square - 5/2(y)
  Input2/1 -> Square - 5/3(z)
  Square - 5/3(F_yy_5) -> F_yy_5/1, Output2/1, Scope/3
  Square - 5/2(F_xz_5) -> F_xz_5/1, Output1/1, Scope/2
  Square - 5/4(F_yz_5) -> F_yz_5/1, Output3/1, Scope/4
  Square - 5/1(F_xx_5) -> F_xx_5/1, Output/1, Scope/1

== system All_64_Coils/Coil-33/Outer_5_Coils/Square-10 ==
  Input : Inport
  Input1 : Inport  Port=2
  Input2 : Inport  Port=3
  Input3 : Inport  Port=4
  F_xx_10 : Display
  F_xz_10 : Display
  F_yy_10 : Display
  F_yz_10 : Display
  Scope : Scope  NumInputPorts=4
  Square - 10 : MATLAB Function  TreatAsAtomicUnit=on
  Output : Outport
  Output1 : Outport  Port=2
  Output2 : Outport  Port=3
  Output3 : Outport  Port=4
  wires:
  Square - 10/1(F_xx_10) -> Output/1, F_xx_10/1, Scope/1
  Square - 10/4(F_yz_10) -> Output3/1, F_yz_10/1, Scope/4
  Square - 10/2(F_xz_10) -> Output1/1, F_xz_10/1, Scope/2
  Square - 10/3(F_yy_10) -> Output2/1, F_yy_10/1, Scope/3
  Input2/1 -> Square - 10/3(z)
  Input1/1 -> Square - 10/2(y)
  Input/1 -> Square - 10/1(x)
  Input3/1 -> Square - 10/4(I)

== system All_64_Coils/Coil-33/Outer_5_Coils/Square-6 ==
  Input : Inport
  Input1 : Inport  Port=2
  Input2 : Inport  Port=3
  Input3 : Inport  Port=4
  F_xx_6 : Display
  F_xz_6 : Display
  F_yy_6 : Display
  F_yz_6 : Display
  Scope : Scope  NumInputPorts=4
  Square - 6 : MATLAB Function  TreatAsAtomicUnit=on
  Output : Outport
  Output1 : Outport  Port=2
  Output2 : Outport  Port=3
  Output3 : Outport  Port=4
  wires:
  Square - 6/2(F_xz_6) -> Output1/1, F_xz_6/1, Scope/2
  Square - 6/3(F_yy_6) -> F_yy_6/1, Output2/1, Scope/3
  Square - 6/1(F_xx_6) -> Output/1, F_xx_6/1, Scope/1
  Square - 6/4(F_yz_6) -> Output3/1, F_yz_6/1, Scope/4
  Input/1 -> Square - 6/1(x)
  Input1/1 -> Square - 6/2(y)
  Input2/1 -> Square - 6/3(z)
  Input3/1 -> Square - 6/4(I)

== system All_64_Coils/Coil-33/Outer_5_Coils/Square-7 ==
  Input : Inport
  Input1 : Inport  Port=2
  Input2 : Inport  Port=3
  Input3 : Inport  Port=4
  F_xx_7 : Display
  F_xz_7 : Display
  F_yy_7 : Display
  F_yz_7 : Display
  Scope : Scope  NumInputPorts=4
  Square - 7 : MATLAB Function  TreatAsAtomicUnit=on
  Output : Outport
  Output1 : Outport  Port=2
  Output2 : Outport  Port=3
  Output3 : Outport  Port=4
  wires:
  Square - 7/1(F_xx_7) -> Output/1, F_xx_7/1, Scope/1
  Square - 7/2(F_xz_7) -> Output1/1, F_xz_7/1, Scope/2
  Square - 7/4(F_yz_7) -> Output3/1, F_yz_7/1, Scope/4
  Square - 7/3(F_yy_7) -> Output2/1, F_yy_7/1, Scope/3
  Input2/1 -> Square - 7/3(z)
  Input1/1 -> Square - 7/2(y)
  Input/1 -> Square - 7/1(x)
  Input3/1 -> Square - 7/4(I)

== system All_64_Coils/Coil-33/Outer_5_Coils/Square-8 ==
  Input : Inport
  Input1 : Inport  Port=2
  Input2 : Inport  Port=3
  Input3 : Inport  Port=4
  F_xx_8 : Display
  F_xz_8 : Display
  F_yy_8 : Display
  F_yz_8 : Display
  Scope : Scope  NumInputPorts=4
  Square - 8 : MATLAB Function  TreatAsAtomicUnit=on
  Output : Outport
  Output1 : Outport  Port=2
  Output2 : Outport  Port=3
  Output3 : Outport  Port=4
  wires:
  Square - 8/1(F_xx_8) -> Output/1, F_xx_8/1, Scope/1
  Square - 8/4(F_yz_8) -> Output3/1, F_yz_8/1, Scope/4
  Square - 8/2(F_xz_8) -> Output1/1, F_xz_8/1, Scope/2
  Square - 8/3(F_yy_8) -> F_yy_8/1, Output2/1, Scope/3
  Input2/1 -> Square - 8/3(z)
  Input1/1 -> Square - 8/2(y)
  Input/1 -> Square - 8/1(x)
  Input3/1 -> Square - 8/4(I)

== system All_64_Coils/Coil-33/Outer_5_Coils/Square-9 ==
  Input : Inport
  Input1 : Inport  Port=2
  Input2 : Inport  Port=3
  Input3 : Inport  Port=4
  F_xx_9 : Display
  F_xz_9 : Display
  F_yy_9 : Display
  F_yz_9 : Display
  Scope : Scope  NumInputPorts=4
  Square - 9 : MATLAB Function  TreatAsAtomicUnit=on
  Output : Outport
  Output1 : Outport  Port=2
  Output2 : Outport  Port=3
  Output3 : Outport  Port=4
  wires:
  Square - 9/1(F_xx_9) -> Output/1, F_xx_9/1, Scope/1
  Square - 9/4(F_yz_9) -> Output3/1, F_yz_9/1, Scope/4
  Square - 9/2(F_xz_9) -> Output1/1, F_xz_9/1, Scope/2
  Square - 9/3(F_yy_9) -> Output2/1, F_yy_9/1, Scope/3
  Input2/1 -> Square - 9/3(z)
  Input1/1 -> Square - 9/2(y)
  Input/1 -> Square - 9/1(x)
  Input3/1 -> Square - 9/4(I)

== system All_64_Coils/Coil-34/Inner_5_coils/Square-1 ==
  Input : Inport
  Input1 : Inport  Port=2
  Input2 : Inport  Port=3
  Input3 : Inport  Port=4
  F_xx_1 : Display
  F_xz_1 : Display
  F_yy_1 : Display
  F_yz_1 : Display
  Scope : Scope  NumInputPorts=4
  Square - 1 : MATLAB Function  TreatAsAtomicUnit=on
  Output : Outport
  Output1 : Outport  Port=2
  Output2 : Outport  Port=3
  Output3 : Outport  Port=4
  wires:
  Input3/1 -> Square - 1/4(I)
  Input2/1 -> Square - 1/3(z)
  Input1/1 -> Square - 1/2(y)
  Input/1 -> Square - 1/1(x)
  Square - 1/4(F_yz_1) -> F_yz_1/1, Output3/1, Scope/4
  Square - 1/1(F_xx_1) -> Scope/1, F_xx_1/1, Output/1
  Square - 1/3(F_yy_1) -> Scope/3, Output2/1, F_yy_1/1
  Square - 1/2(F_xz_1) -> Scope/2, F_xz_1/1, Output1/1

== system All_64_Coils/Coil-34/Inner_5_coils/Square-2 ==
  Input : Inport
  Input1 : Inport  Port=2
  Input2 : Inport  Port=3
  Input3 : Inport  Port=4
  F_xx_2 : Display
  F_xz_2 : Display
  F_yy_2 : Display
  F_yz_2 : Display
  Scope : Scope  NumInputPorts=4
  Square - 2 : MATLAB Function  TreatAsAtomicUnit=on
  Output : Outport
  Output1 : Outport  Port=2
  Output2 : Outport  Port=3
  Output3 : Outport  Port=4
  wires:
  Input3/1 -> Square - 2/4(I)
  Input/1 -> Square - 2/1(x)
  Input1/1 -> Square - 2/2(y)
  Input2/1 -> Square - 2/3(z)
  Square - 2/3(F_yy_2) -> F_yy_2/1, Output2/1, Scope/3
  Square - 2/4(F_yz_2) -> F_yz_2/1, Output3/1, Scope/4
  Square - 2/2(F_xz_2) -> F_xz_2/1, Output1/1, Scope/2
  Square - 2/1(F_xx_2) -> F_xx_2/1, Output/1, Scope/1

== system All_64_Coils/Coil-34/Inner_5_coils/Square-3 ==
  Input : Inport
  Input1 : Inport  Port=2
  Input2 : Inport  Port=3
  Input3 : Inport  Port=4
  F_xx_3 : Display
  F_xz_3 : Display
  F_yy_3 : Display
  F_yz_3 : Display
  Scope : Scope  NumInputPorts=4
  Square - 3 : MATLAB Function  TreatAsAtomicUnit=on
  Output : Outport
  Output1 : Outport  Port=2
  Output2 : Outport  Port=3
  Output3 : Outport  Port=4
  wires:
  Input3/1 -> Square - 3/4(I)
  Input/1 -> Square - 3/1(x)
  Input1/1 -> Square - 3/2(y)
  Input2/1 -> Square - 3/3(z)
  Square - 3/3(F_yy_3) -> Output2/1, F_yy_3/1, Scope/3
  Square - 3/2(F_xz_3) -> F_xz_3/1, Output1/1, Scope/2
  Square - 3/4(F_yz_3) -> F_yz_3/1, Output3/1, Scope/4
  Square - 3/1(F_xx_3) -> F_xx_3/1, Output/1, Scope/1

== system All_64_Coils/Coil-34/Inner_5_coils/Square-4 ==
  Input : Inport
  Input1 : Inport  Port=2
  Input2 : Inport  Port=3
  Input3 : Inport  Port=4
  F_xx_4 : Display
  F_xz_4 : Display
  F_yy_4 : Display
  F_yz_4 : Display
  Scope : Scope  NumInputPorts=4
  Square - 4 : MATLAB Function  TreatAsAtomicUnit=on
  Output : Outport
  Output1 : Outport  Port=2
  Output2 : Outport  Port=3
  Output3 : Outport  Port=4
  wires:
  Input3/1 -> Square - 4/4(I)
  Input/1 -> Square - 4/1(x)
  Input1/1 -> Square - 4/2(y)
  Input2/1 -> Square - 4/3(z)
  Square - 4/3(F_yy_4) -> F_yy_4/1, Output2/1, Scope/3
  Square - 4/2(F_xz_4) -> Scope/2, F_xz_4/1, Output1/1
  Square - 4/4(F_yz_4) -> F_yz_4/1, Output3/1, Scope/4
  Square - 4/1(F_xx_4) -> F_xx_4/1, Output/1, Scope/1

== system All_64_Coils/Coil-34/Inner_5_coils/Square-5 ==
  Input : Inport
  Input1 : Inport  Port=2
  Input2 : Inport  Port=3
  Input3 : Inport  Port=4
  F_xx_5 : Display
  F_xz_5 : Display
  F_yy_5 : Display
  F_yz_5 : Display
  Scope : Scope  NumInputPorts=4
  Square - 5 : MATLAB Function  TreatAsAtomicUnit=on
  Output : Outport
  Output1 : Outport  Port=2
  Output2 : Outport  Port=3
  Output3 : Outport  Port=4
  wires:
  Input3/1 -> Square - 5/4(I)
  Input/1 -> Square - 5/1(x)
  Input1/1 -> Square - 5/2(y)
  Input2/1 -> Square - 5/3(z)
  Square - 5/3(F_yy_5) -> F_yy_5/1, Output2/1, Scope/3
  Square - 5/2(F_xz_5) -> F_xz_5/1, Output1/1, Scope/2
  Square - 5/4(F_yz_5) -> F_yz_5/1, Output3/1, Scope/4
  Square - 5/1(F_xx_5) -> F_xx_5/1, Output/1, Scope/1

== system All_64_Coils/Coil-34/Outer_5_Coils/Square-10 ==
  Input : Inport
  Input1 : Inport  Port=2
  Input2 : Inport  Port=3
  Input3 : Inport  Port=4
  F_xx_10 : Display
  F_xz_10 : Display
  F_yy_10 : Display
  F_yz_10 : Display
  Scope : Scope  NumInputPorts=4
  Square - 10 : MATLAB Function  TreatAsAtomicUnit=on
  Output : Outport
  Output1 : Outport  Port=2
  Output2 : Outport  Port=3
  Output3 : Outport  Port=4
  wires:
  Square - 10/1(F_xx_10) -> Output/1, F_xx_10/1, Scope/1
  Square - 10/4(F_yz_10) -> Output3/1, F_yz_10/1, Scope/4
  Square - 10/2(F_xz_10) -> Output1/1, F_xz_10/1, Scope/2
  Square - 10/3(F_yy_10) -> Output2/1, F_yy_10/1, Scope/3
  Input2/1 -> Square - 10/3(z)
  Input1/1 -> Square - 10/2(y)
  Input/1 -> Square - 10/1(x)
  Input3/1 -> Square - 10/4(I)

== system All_64_Coils/Coil-34/Outer_5_Coils/Square-6 ==
  Input : Inport
  Input1 : Inport  Port=2
  Input2 : Inport  Port=3
  Input3 : Inport  Port=4
  F_xx_6 : Display
  F_xz_6 : Display
  F_yy_6 : Display
  F_yz_6 : Display
  Scope : Scope  NumInputPorts=4
  Square - 6 : MATLAB Function  TreatAsAtomicUnit=on
  Output : Outport
  Output1 : Outport  Port=2
  Output2 : Outport  Port=3
  Output3 : Outport  Port=4
  wires:
  Square - 6/2(F_xz_6) -> Output1/1, F_xz_6/1, Scope/2
  Square - 6/3(F_yy_6) -> F_yy_6/1, Output2/1, Scope/3
  Square - 6/1(F_xx_6) -> Output/1, F_xx_6/1, Scope/1
  Square - 6/4(F_yz_6) -> Output3/1, F_yz_6/1, Scope/4
  Input/1 -> Square - 6/1(x)
  Input1/1 -> Square - 6/2(y)
  Input2/1 -> Square - 6/3(z)
  Input3/1 -> Square - 6/4(I)

== system All_64_Coils/Coil-34/Outer_5_Coils/Square-7 ==
  Input : Inport
  Input1 : Inport  Port=2
  Input2 : Inport  Port=3
  Input3 : Inport  Port=4
  F_xx_7 : Display
  F_xz_7 : Display
  F_yy_7 : Display
  F_yz_7 : Display
  Scope : Scope  NumInputPorts=4
  Square - 7 : MATLAB Function  TreatAsAtomicUnit=on
  Output : Outport
  Output1 : Outport  Port=2
  Output2 : Outport  Port=3
  Output3 : Outport  Port=4
  wires:
  Square - 7/1(F_xx_7) -> Output/1, F_xx_7/1, Scope/1
  Square - 7/2(F_xz_7) -> Output1/1, F_xz_7/1, Scope/2
  Square - 7/4(F_yz_7) -> Output3/1, F_yz_7/1, Scope/4
  Square - 7/3(F_yy_7) -> Output2/1, F_yy_7/1, Scope/3
  Input2/1 -> Square - 7/3(z)
  Input1/1 -> Square - 7/2(y)
  Input/1 -> Square - 7/1(x)
  Input3/1 -> Square - 7/4(I)

== system All_64_Coils/Coil-34/Outer_5_Coils/Square-8 ==
  Input : Inport
  Input1 : Inport  Port=2
  Input2 : Inport  Port=3
  Input3 : Inport  Port=4
  F_xx_8 : Display
  F_xz_8 : Display
  F_yy_8 : Display
  F_yz_8 : Display
  Scope : Scope  NumInputPorts=4
  Square - 8 : MATLAB Function  TreatAsAtomicUnit=on
  Output : Outport
  Output1 : Outport  Port=2
  Output2 : Outport  Port=3
  Output3 : Outport  Port=4
  wires:
  Square - 8/1(F_xx_8) -> Output/1, F_xx_8/1, Scope/1
  Square - 8/4(F_yz_8) -> Output3/1, F_yz_8/1, Scope/4
  Square - 8/2(F_xz_8) -> Output1/1, F_xz_8/1, Scope/2
  Square - 8/3(F_yy_8) -> F_yy_8/1, Output2/1, Scope/3
  Input2/1 -> Square - 8/3(z)
  Input1/1 -> Square - 8/2(y)
  Input/1 -> Square - 8/1(x)
  Input3/1 -> Square - 8/4(I)

== system All_64_Coils/Coil-34/Outer_5_Coils/Square-9 ==
  Input : Inport
  Input1 : Inport  Port=2
  Input2 : Inport  Port=3
  Input3 : Inport  Port=4
  F_xx_9 : Display
  F_xz_9 : Display
  F_yy_9 : Display
  F_yz_9 : Display
  Scope : Scope  NumInputPorts=4
  Square - 9 : MATLAB Function  TreatAsAtomicUnit=on
  Output : Outport
  Output1 : Outport  Port=2
  Output2 : Outport  Port=3
  Output3 : Outport  Port=4
  wires:
  Square - 9/1(F_xx_9) -> Output/1, F_xx_9/1, Scope/1
  Square - 9/4(F_yz_9) -> Output3/1, F_yz_9/1, Scope/4
  Square - 9/2(F_xz_9) -> Output1/1, F_xz_9/1, Scope/2
  Square - 9/3(F_yy_9) -> Output2/1, F_yy_9/1, Scope/3
  Input2/1 -> Square - 9/3(z)
  Input1/1 -> Square - 9/2(y)
  Input/1 -> Square - 9/1(x)
  Input3/1 -> Square - 9/4(I)

== system All_64_Coils/Coil-35/Inner_5_coils/Square-1 ==
  Input : Inport
  Input1 : Inport  Port=2
  Input2 : Inport  Port=3
  Input3 : Inport  Port=4
  F_xx_1 : Display
  F_xz_1 : Display
  F_yy_1 : Display
  F_yz_1 : Display
  Scope : Scope  NumInputPorts=4
  Square - 1 : MATLAB Function  TreatAsAtomicUnit=on
  Output : Outport
  Output1 : Outport  Port=2
  Output2 : Outport  Port=3
  Output3 : Outport  Port=4
  wires:
  Input3/1 -> Square - 1/4(I)
  Input2/1 -> Square - 1/3(z)
  Input1/1 -> Square - 1/2(y)
  Input/1 -> Square - 1/1(x)
  Square - 1/4(F_yz_1) -> F_yz_1/1, Output3/1, Scope/4
  Square - 1/1(F_xx_1) -> Scope/1, F_xx_1/1, Output/1
  Square - 1/3(F_yy_1) -> Scope/3, Output2/1, F_yy_1/1
  Square - 1/2(F_xz_1) -> Scope/2, F_xz_1/1, Output1/1

== system All_64_Coils/Coil-35/Inner_5_coils/Square-2 ==
  Input : Inport
  Input1 : Inport  Port=2
  Input2 : Inport  Port=3
  Input3 : Inport  Port=4
  F_xx_2 : Display
  F_xz_2 : Display
  F_yy_2 : Display
  F_yz_2 : Display
  Scope : Scope  NumInputPorts=4
  Square - 2 : MATLAB Function  TreatAsAtomicUnit=on
  Output : Outport
  Output1 : Outport  Port=2
  Output2 : Outport  Port=3
  Output3 : Outport  Port=4
  wires:
  Input3/1 -> Square - 2/4(I)
  Input/1 -> Square - 2/1(x)
  Input1/1 -> Square - 2/2(y)
  Input2/1 -> Square - 2/3(z)
  Square - 2/3(F_yy_2) -> F_yy_2/1, Output2/1, Scope/3
  Square - 2/4(F_yz_2) -> F_yz_2/1, Output3/1, Scope/4
  Square - 2/2(F_xz_2) -> F_xz_2/1, Output1/1, Scope/2
  Square - 2/1(F_xx_2) -> F_xx_2/1, Output/1, Scope/1

== system All_64_Coils/Coil-35/Inner_5_coils/Square-3 ==
  Input : Inport
  Input1 : Inport  Port=2
  Input2 : Inport  Port=3
  Input3 : Inport  Port=4
  F_xx_3 : Display
  F_xz_3 : Display
  F_yy_3 : Display
  F_yz_3 : Display
  Scope : Scope  NumInputPorts=4
  Square - 3 : MATLAB Function  TreatAsAtomicUnit=on
  Output : Outport
  Output1 : Outport  Port=2
  Output2 : Outport  Port=3
  Output3 : Outport  Port=4
  wires:
  Input3/1 -> Square - 3/4(I)
  Input/1 -> Square - 3/1(x)
  Input1/1 -> Square - 3/2(y)
  Input2/1 -> Square - 3/3(z)
  Square - 3/3(F_yy_3) -> Output2/1, F_yy_3/1, Scope/3
  Square - 3/2(F_xz_3) -> F_xz_3/1, Output1/1, Scope/2
  Square - 3/4(F_yz_3) -> F_yz_3/1, Output3/1, Scope/4
  Square - 3/1(F_xx_3) -> F_xx_3/1, Output/1, Scope/1

== system All_64_Coils/Coil-35/Inner_5_coils/Square-4 ==
  Input : Inport
  Input1 : Inport  Port=2
  Input2 : Inport  Port=3
  Input3 : Inport  Port=4
  F_xx_4 : Display
  F_xz_4 : Display
  F_yy_4 : Display
  F_yz_4 : Display
  Scope : Scope  NumInputPorts=4
  Square - 4 : MATLAB Function  TreatAsAtomicUnit=on
  Output : Outport
  Output1 : Outport  Port=2
  Output2 : Outport  Port=3
  Output3 : Outport  Port=4
  wires:
  Input3/1 -> Square - 4/4(I)
  Input/1 -> Square - 4/1(x)
  Input1/1 -> Square - 4/2(y)
  Input2/1 -> Square - 4/3(z)
  Square - 4/3(F_yy_4) -> F_yy_4/1, Output2/1, Scope/3
  Square - 4/2(F_xz_4) -> Scope/2, F_xz_4/1, Output1/1
  Square - 4/4(F_yz_4) -> F_yz_4/1, Output3/1, Scope/4
  Square - 4/1(F_xx_4) -> F_xx_4/1, Output/1, Scope/1

== system All_64_Coils/Coil-35/Inner_5_coils/Square-5 ==
  Input : Inport
  Input1 : Inport  Port=2
  Input2 : Inport  Port=3
  Input3 : Inport  Port=4
  F_xx_5 : Display
  F_xz_5 : Display
  F_yy_5 : Display
  F_yz_5 : Display
  Scope : Scope  NumInputPorts=4
  Square - 5 : MATLAB Function  TreatAsAtomicUnit=on
  Output : Outport
  Output1 : Outport  Port=2
  Output2 : Outport  Port=3
  Output3 : Outport  Port=4
  wires:
  Input3/1 -> Square - 5/4(I)
  Input/1 -> Square - 5/1(x)
  Input1/1 -> Square - 5/2(y)
  Input2/1 -> Square - 5/3(z)
  Square - 5/3(F_yy_5) -> F_yy_5/1, Output2/1, Scope/3
  Square - 5/2(F_xz_5) -> F_xz_5/1, Output1/1, Scope/2
  Square - 5/4(F_yz_5) -> F_yz_5/1, Output3/1, Scope/4
  Square - 5/1(F_xx_5) -> F_xx_5/1, Output/1, Scope/1

== system All_64_Coils/Coil-35/Outer_5_Coils/Square-10 ==
  Input : Inport
  Input1 : Inport  Port=2
  Input2 : Inport  Port=3
  Input3 : Inport  Port=4
  F_xx_10 : Display
  F_xz_10 : Display
  F_yy_10 : Display
  F_yz_10 : Display
  Scope : Scope  NumInputPorts=4
  Square - 10 : MATLAB Function  TreatAsAtomicUnit=on
  Output : Outport
  Output1 : Outport  Port=2
  Output2 : Outport  Port=3
  Output3 : Outport  Port=4
  wires:
  Square - 10/1(F_xx_10) -> Output/1, F_xx_10/1, Scope/1
  Square - 10/4(F_yz_10) -> Output3/1, F_yz_10/1, Scope/4
  Square - 10/2(F_xz_10) -> Output1/1, F_xz_10/1, Scope/2
  Square - 10/3(F_yy_10) -> Output2/1, F_yy_10/1, Scope/3
  Input2/1 -> Square - 10/3(z)
  Input1/1 -> Square - 10/2(y)
  Input/1 -> Square - 10/1(x)
  Input3/1 -> Square - 10/4(I)

== system All_64_Coils/Coil-35/Outer_5_Coils/Square-6 ==
  Input : Inport
  Input1 : Inport  Port=2
  Input2 : Inport  Port=3
  Input3 : Inport  Port=4
  F_xx_6 : Display
  F_xz_6 : Display
  F_yy_6 : Display
  F_yz_6 : Display
  Scope : Scope  NumInputPorts=4
  Square - 6 : MATLAB Function  TreatAsAtomicUnit=on
  Output : Outport
  Output1 : Outport  Port=2
  Output2 : Outport  Port=3
  Output3 : Outport  Port=4
  wires:
  Square - 6/2(F_xz_6) -> Output1/1, F_xz_6/1, Scope/2
  Square - 6/3(F_yy_6) -> F_yy_6/1, Output2/1, Scope/3
  Square - 6/1(F_xx_6) -> Output/1, F_xx_6/1, Scope/1
  Square - 6/4(F_yz_6) -> Output3/1, F_yz_6/1, Scope/4
  Input/1 -> Square - 6/1(x)
  Input1/1 -> Square - 6/2(y)
  Input2/1 -> Square - 6/3(z)
  Input3/1 -> Square - 6/4(I)
